simulink model "LoFT_Example2"  (R2022b)
settings: Solver=ode4  StartTime=0.0  StopTime=300  FixedStep=simu.dt  RelTol=1e-3
PreLoadFcn:
  | simu.dt = 0.025;
  | simu.Tmax = 600;
undefined symbols (not defined in the file): body, circtime, Communication, irkb, LoFT, Turbwind, waves, wind
libraries: simulink x350
(part 46 of 46)

== matlab function LoFT_Example2/FOWT System/Subsystem5/FOWT3/Hydrodynamics/radiation force/MATLAB Function ==
  data: velocity input; radiationdamping output; irkb input; circtime input
function radiationdamping  = fcn(velocity,irkb,circtime)

radiationdamping  = ConvolutionIntegral_interp(velocity,irkb,circtime);
end

== matlab function LoFT_Example2/FOWT System/Subsystem5/FOWT4/Hydrodynamics/excitation force/WECSim Linear wave excitiation ==
  data: A input; Fext output; w input; fExtRE input; fExtIM input; phaseRand input; dw input; time input; WaveDir input; spread input; fExtMD input
function Fext = irregExcF(A,w,fExtRE,fExtIM,phaseRand,dw,time,WaveDir,spread,fExtMD)

Fext = irregExcF(A,w,fExtRE,fExtIM,phaseRand,dw,time,WaveDir,spread,fExtMD);

end

== matlab function LoFT_Example2/FOWT System/Subsystem5/FOWT4/Hydrodynamics/radiation force/MATLAB Function ==
  data: velocity input; radiationdamping output; irkb input; circtime input
function radiationdamping  = fcn(velocity,irkb,circtime)

radiationdamping  = ConvolutionIntegral_interp(velocity,irkb,circtime);
end

== matlab function LoFT_Example2/FOWT System/Subsystem5/FOWT5/Hydrodynamics/excitation force/WECSim Linear wave excitiation ==
  data: A input; Fext output; w input; fExtRE input; fExtIM input; phaseRand input; dw input; time input; WaveDir input; spread input; fExtMD input
function Fext = irregExcF(A,w,fExtRE,fExtIM,phaseRand,dw,time,WaveDir,spread,fExtMD)

Fext = irregExcF(A,w,fExtRE,fExtIM,phaseRand,dw,time,WaveDir,spread,fExtMD);

end

== matlab function LoFT_Example2/FOWT System/Subsystem5/FOWT5/Hydrodynamics/radiation force/MATLAB Function ==
  data: velocity input; radiationdamping output; irkb input; circtime input
function radiationdamping  = fcn(velocity,irkb,circtime)

radiationdamping  = ConvolutionIntegral_interp(velocity,irkb,circtime);
end

== matlab function LoFT_Example2/FOWT System/Subsystem5/FOWT6/Hydrodynamics/excitation force/WECSim Linear wave excitiation ==
  data: A input; Fext output; w input; fExtRE input; fExtIM input; phaseRand input; dw input; time input; WaveDir input; spread input; fExtMD input
function Fext = irregExcF(A,w,fExtRE,fExtIM,phaseRand,dw,time,WaveDir,spread,fExtMD)

Fext = irregExcF(A,w,fExtRE,fExtIM,phaseRand,dw,time,WaveDir,spread,fExtMD);

end

== matlab function LoFT_Example2/FOWT System/Subsystem5/FOWT6/Hydrodynamics/radiation force/MATLAB Function ==
  data: velocity input; radiationdamping output; irkb input; circtime input
function radiationdamping  = fcn(velocity,irkb,circtime)

radiationdamping  = ConvolutionIntegral_interp(velocity,irkb,circtime);
end

== matlab function LoFT_Example2/FOWT System/Subsystem1/FOWT5/Hydrodynamics/excitation force/WECSim Linear wave excitiation ==
  data: A input; Fext output; w input; fExtRE input; fExtIM input; phaseRand input; dw input; time input; WaveDir input; spread input; fExtMD input
function Fext = irregExcF(A,w,fExtRE,fExtIM,phaseRand,dw,time,WaveDir,spread,fExtMD)

Fext = irregExcF(A,w,fExtRE,fExtIM,phaseRand,dw,time,WaveDir,spread,fExtMD);

end

== matlab function LoFT_Example2/FOWT System/Subsystem5/FOWT7/Hydrodynamics/excitation force/WECSim Linear wave excitiation ==
  data: A input; Fext output; w input; fExtRE input; fExtIM input; phaseRand input; dw input; time input; WaveDir input; spread input; fExtMD input
function Fext = irregExcF(A,w,fExtRE,fExtIM,phaseRand,dw,time,WaveDir,spread,fExtMD)

Fext = irregExcF(A,w,fExtRE,fExtIM,phaseRand,dw,time,WaveDir,spread,fExtMD);

end

== matlab function LoFT_Example2/FOWT System/Subsystem5/FOWT7/Hydrodynamics/radiation force/MATLAB Function ==
  data: velocity input; radiationdamping output; irkb input; circtime input
function radiationdamping  = fcn(velocity,irkb,circtime)

radiationdamping  = ConvolutionIntegral_interp(velocity,irkb,circtime);
end

== matlab function LoFT_Example2/FOWT System/Subsystem5/FOWT8/Hydrodynamics/excitation force/WECSim Linear wave excitiation ==
  data: A input; Fext output; w input; fExtRE input; fExtIM input; phaseRand input; dw input; time input; WaveDir input; spread input; fExtMD input
function Fext = irregExcF(A,w,fExtRE,fExtIM,phaseRand,dw,time,WaveDir,spread,fExtMD)

Fext = irregExcF(A,w,fExtRE,fExtIM,phaseRand,dw,time,WaveDir,spread,fExtMD);

end

== matlab function LoFT_Example2/FOWT System/Subsystem5/FOWT8/Hydrodynamics/radiation force/MATLAB Function ==
  data: velocity input; radiationdamping output; irkb input; circtime input
function radiationdamping  = fcn(velocity,irkb,circtime)

radiationdamping  = ConvolutionIntegral_interp(velocity,irkb,circtime);
end

== matlab function LoFT_Example2/FOWT System/Subsystem5/FOWT9/Hydrodynamics/excitation force/WECSim Linear wave excitiation ==
  data: A input; Fext output; w input; fExtRE input; fExtIM input; phaseRand input; dw input; time input; WaveDir input; spread input; fExtMD input
function Fext = irregExcF(A,w,fExtRE,fExtIM,phaseRand,dw,time,WaveDir,spread,fExtMD)

Fext = irregExcF(A,w,fExtRE,fExtIM,phaseRand,dw,time,WaveDir,spread,fExtMD);

end

== matlab function LoFT_Example2/FOWT System/Subsystem5/FOWT9/Hydrodynamics/radiation force/MATLAB Function ==
  data: velocity input; radiationdamping output; irkb input; circtime input
function radiationdamping  = fcn(velocity,irkb,circtime)

radiationdamping  = ConvolutionIntegral_interp(velocity,irkb,circtime);
end

== matlab function LoFT_Example2/FOWT System/Subsystem7/FOWT1/Hydrodynamics/excitation force/WECSim Linear wave excitiation ==
  data: A input; Fext output; w input; fExtRE input; fExtIM input; phaseRand input; dw input; time input; WaveDir input; spread input; fExtMD input
function Fext = irregExcF(A,w,fExtRE,fExtIM,phaseRand,dw,time,WaveDir,spread,fExtMD)

Fext = irregExcF(A,w,fExtRE,fExtIM,phaseRand,dw,time,WaveDir,spread,fExtMD);

end
